simulink model "cddDcmApp"  (R2022b)
settings: Solver=FixedStepDiscrete  StartTime=0.0  StopTime=10.0  FixedStep=0.02  RelTol=1e-3
undefined symbols (not defined in the file or in its folder): CCPFaultType, DcChlType, HFAChlType, IcuChannelType, MtrRoutStaEnum, P_SignalPartType, P_Std_ReturnType, PowerChlType, SrsAdChlType, VGAChlType
(part 13 of 13)

== system cddDcmApp/DataServices_DID_D986_Vent_Grill_Actuator12_Second_Right_Y_Flap_DID_D986_2_No_Of_Movements/DataServices_DID_D986_Vent_Grill_Actuator12_Second_Right_Y_Flap_DID_D986_2_No_Of_Movements_WriteData_sys ==
  function : TriggerPort  TriggerType=function-call  IsSimulinkFunction=on  FunctionName=DataServices_DID_D986_Vent_Grill_Actuator12_Second_Right_Y_Flap_DID_D986_2_No_Of_Movements_WriteData  VariantControl=(inherit)
  Ground_1 : Ground
  Ground_2 : Ground
  Terminator_1 : Terminator
  Terminator_2 : Terminator
  Data : ArgIn  OutDataTypeStr=uint8  PortDimensions=4  ArgumentName=Data
  OpStatus : ArgIn  Port=2  OutDataTypeStr=Enum: Dcm_OpStatusType  ArgumentName=OpStatus
  ErrorCode : ArgOut  OutDataTypeStr=Enum: Dcm_NegativeResponseCodeType  ArgumentName=ErrorCode
  ERR : ArgOut  Port=2  OutDataTypeStr=Std_ReturnType  ArgumentName=ERR
  wires:
  Data/1 -> Terminator_1/1
  OpStatus/1 -> Terminator_2/1
  Ground_1/1 -> ErrorCode/1
  Ground_2/1 -> ERR/1

== system cddDcmApp/DataServices_DID_D987_Vent_Grill_Actuator12_Second_Right_Y_Flap_position_ComplexStructure_2/DataServices_DID_D987_Vent_Grill_Actuator12_Second_Right_Y_Flap_position_ComplexStructure_2_ConditionCheckRead_sys ==
  function : TriggerPort  TriggerType=function-call  IsSimulinkFunction=on  FunctionName=DataServices_DID_D987_Vent_Grill_Actuator12_Second_Right_Y_Flap_position_ComplexStructure_2_ConditionCheckRead  VariantControl=(inherit)
  Ground_1 : Ground
  Ground_2 : Ground
  ErrorCode : ArgOut  OutDataTypeStr=Enum: Dcm_NegativeResponseCodeType  ArgumentName=ErrorCode
  ERR : ArgOut  Port=2  OutDataTypeStr=Std_ReturnType  ArgumentName=ERR
  wires:
  Ground_1/1 -> ErrorCode/1
  Ground_2/1 -> ERR/1

== system cddDcmApp/DataServices_DID_D987_Vent_Grill_Actuator12_Second_Right_Y_Flap_position_ComplexStructure_2/DataServices_DID_D987_Vent_Grill_Actuator12_Second_Right_Y_Flap_position_ComplexStructure_2_ReadData_sys ==
  function : TriggerPort  TriggerType=function-call  IsSimulinkFunction=on  FunctionName=DataServices_DID_D987_Vent_Grill_Actuator12_Second_Right_Y_Flap_position_ComplexStructure_2_ReadData  VariantControl=(inherit)
  Ground_1 : Ground
  Ground_2 : Ground
  Data : ArgOut  OutDataTypeStr=uint8  ArgumentName=Data
  ERR : ArgOut  Port=2  OutDataTypeStr=Std_ReturnType  ArgumentName=ERR
  wires:
  Ground_1/1 -> Data/1
  Ground_2/1 -> ERR/1

== system cddDcmApp/DataServices_DID_D987_Vent_Grill_Actuator12_Second_Right_Y_Flap_position_DID_D987_1_Requested_Position/DataServices_DID_D987_Vent_Grill_Actuator12_Second_Right_Y_Flap_position_DID_D987_1_Requested_Position_ConditionCheckRead_sys ==
  function : TriggerPort  TriggerType=function-call  IsSimulinkFunction=on  FunctionName=<binary blob, 121 chars, not shown>  VariantControl=(inherit)
  Ground_1 : Ground
  Ground_2 : Ground
  ErrorCode : ArgOut  OutDataTypeStr=Enum: Dcm_NegativeResponseCodeType  ArgumentName=ErrorCode
  ERR : ArgOut  Port=2  OutDataTypeStr=Std_ReturnType  ArgumentName=ERR
  wires:
  Ground_1/1 -> ErrorCode/1
  Ground_2/1 -> ERR/1

== system cddDcmApp/DataServices_DID_D987_Vent_Grill_Actuator12_Second_Right_Y_Flap_position_DID_D987_1_Requested_Position/DataServices_DID_D987_Vent_Grill_Actuator12_Second_Right_Y_Flap_position_DID_D987_1_Requested_Position_ReadData_sys ==
  function : TriggerPort  TriggerType=function-call  IsSimulinkFunction=on  FunctionName=DataServices_DID_D987_Vent_Grill_Actuator12_Second_Right_Y_Flap_position_DID_D987_1_Requested_Position_ReadData  VariantControl=(inherit)
  Ground_1 : Ground
  Ground_2 : Ground
  Data : ArgOut  OutDataTypeStr=uint8  ArgumentName=Data
  ERR : ArgOut  Port=2  OutDataTypeStr=Std_ReturnType  ArgumentName=ERR
  wires:
  Ground_1/1 -> Data/1
  Ground_2/1 -> ERR/1

== system cddDcmApp/DataServices_DID_D987_Vent_Grill_Actuator12_Second_Right_Y_Flap_position_DID_D987_2_Actual_Position/DataServices_DID_D987_Vent_Grill_Actuator12_Second_Right_Y_Flap_position_DID_D987_2_Actual_Position_ConditionCheckRead_sys ==
  function : TriggerPort  TriggerType=function-call  IsSimulinkFunction=on  FunctionName=DataServices_DID_D987_Vent_Grill_Actuator12_Second_Right_Y_Flap_position_DID_D987_2_Actual_Position_ConditionCheckRead  VariantControl=(inherit)
  Ground_1 : Ground
  Ground_2 : Ground
  ErrorCode : ArgOut  OutDataTypeStr=Enum: Dcm_NegativeResponseCodeType  ArgumentName=ErrorCode
  ERR : ArgOut  Port=2  OutDataTypeStr=Std_ReturnType  ArgumentName=ERR
  wires:
  Ground_1/1 -> ErrorCode/1
  Ground_2/1 -> ERR/1

== system cddDcmApp/DataServices_DID_D987_Vent_Grill_Actuator12_Second_Right_Y_Flap_position_DID_D987_2_Actual_Position/DataServices_DID_D987_Vent_Grill_Actuator12_Second_Right_Y_Flap_position_DID_D987_2_Actual_Position_ReadData_sys ==
  function : TriggerPort  TriggerType=function-call  IsSimulinkFunction=on  FunctionName=DataServices_DID_D987_Vent_Grill_Actuator12_Second_Right_Y_Flap_position_DID_D987_2_Actual_Position_ReadData  VariantControl=(inherit)
  Ground_1 : Ground
  Ground_2 : Ground
  Data : ArgOut  OutDataTypeStr=uint8  ArgumentName=Data
  ERR : ArgOut  Port=2  OutDataTypeStr=Std_ReturnType  ArgumentName=ERR
  wires:
  Ground_1/1 -> Data/1
  Ground_2/1 -> ERR/1

== system cddDcmApp/DataServices_DID_D988_Vent_Grill_Actuator13_Second_Right_Y_Flap_DID_D988_1_step_Range/DataServices_DID_D988_Vent_Grill_Actuator13_Second_Right_Y_Flap_DID_D988_1_step_Range_ReadData_sys ==
  function : TriggerPort  TriggerType=function-call  IsSimulinkFunction=on  FunctionName=DataServices_DID_D988_Vent_Grill_Actuator13_Second_Right_Y_Flap_DID_D988_1_step_Range_ReadData  VariantControl=(inherit)
  Ground_1 : Ground
  Ground_2 : Ground
  Data : ArgOut  OutDataTypeStr=uint8  ArgumentName=Data
  ERR : ArgOut  Port=2  OutDataTypeStr=Std_ReturnType  ArgumentName=ERR
  wires:
  Ground_1/1 -> Data/1
  Ground_2/1 -> ERR/1

== system cddDcmApp/DataServices_DID_D988_Vent_Grill_Actuator13_Second_Right_Y_Flap_DID_D988_1_step_Range/DataServices_DID_D988_Vent_Grill_Actuator13_Second_Right_Y_Flap_DID_D988_1_step_Range_WriteData_sys ==
  function : TriggerPort  TriggerType=function-call  IsSimulinkFunction=on  FunctionName=DataServices_DID_D988_Vent_Grill_Actuator13_Second_Right_Y_Flap_DID_D988_1_step_Range_WriteData  VariantControl=(inherit)
  Ground_1 : Ground
  Ground_2 : Ground
  Terminator_1 : Terminator
  Data : ArgIn  OutDataTypeStr=uint8  PortDimensions=2  ArgumentName=Data
  ErrorCode : ArgOut  OutDataTypeStr=Enum: Dcm_NegativeResponseCodeType  ArgumentName=ErrorCode
  ERR : ArgOut  Port=2  OutDataTypeStr=Std_ReturnType  ArgumentName=ERR
  wires:
  Data/1 -> Terminator_1/1
  Ground_1/1 -> ErrorCode/1
  Ground_2/1 -> ERR/1

== system cddDcmApp/DataServices_DID_D988_Vent_Grill_Actuator13_Second_Right_Y_Flap_DID_D988_2_No_Of_Movements/DataServices_DID_D988_Vent_Grill_Actuator13_Second_Right_Y_Flap_DID_D988_2_No_Of_Movements_ReadData_sys ==
  function : TriggerPort  TriggerType=function-call  IsSimulinkFunction=on  FunctionName=DataServices_DID_D988_Vent_Grill_Actuator13_Second_Right_Y_Flap_DID_D988_2_No_Of_Movements_ReadData  VariantControl=(inherit)
  Ground_1 : Ground
  Ground_2 : Ground
  Data : ArgOut  OutDataTypeStr=uint8  ArgumentName=Data
  ERR : ArgOut  Port=2  OutDataTypeStr=Std_ReturnType  ArgumentName=ERR
  wires:
  Ground_1/1 -> Data/1
  Ground_2/1 -> ERR/1

== system cddDcmApp/DataServices_DID_D988_Vent_Grill_Actuator13_Second_Right_Y_Flap_DID_D988_2_No_Of_Movements/DataServices_DID_D988_Vent_Grill_Actuator13_Second_Right_Y_Flap_DID_D988_2_No_Of_Movements_WriteData_sys ==
  function : TriggerPort  TriggerType=function-call  IsSimulinkFunction=on  FunctionName=DataServices_DID_D988_Vent_Grill_Actuator13_Second_Right_Y_Flap_DID_D988_2_No_Of_Movements_WriteData  VariantControl=(inherit)
  Ground_1 : Ground
  Ground_2 : Ground
  Terminator_1 : Terminator
  Data : ArgIn  OutDataTypeStr=uint8  PortDimensions=4  ArgumentName=Data
  ErrorCode : ArgOut  OutDataTypeStr=Enum: Dcm_NegativeResponseCodeType  ArgumentName=ErrorCode
  ERR : ArgOut  Port=2  OutDataTypeStr=Std_ReturnType  ArgumentName=ERR
  wires:
  Data/1 -> Terminator_1/1
  Ground_1/1 -> ErrorCode/1
  Ground_2/1 -> ERR/1

== system cddDcmApp/DataServices_DID_D989_Vent_Grill_Actuator13_Second_Right_Y_Flap_position_DID_D989_1_Requested_Position/DataServices_DID_D989_Vent_Grill_Actuator13_Second_Right_Y_Flap_position_DID_D989_1_Requested_Position_ReadData_sys ==
  function : TriggerPort  TriggerType=function-call  IsSimulinkFunction=on  FunctionName=DataServices_DID_D989_Vent_Grill_Actuator13_Second_Right_Y_Flap_position_DID_D989_1_Requested_Position_ReadData  VariantControl=(inherit)
  Ground_1 : Ground
  Ground_2 : Ground
  Data : ArgOut  OutDataTypeStr=uint8  ArgumentName=Data
  ERR : ArgOut  Port=2  OutDataTypeStr=Std_ReturnType  ArgumentName=ERR
  wires:
  Ground_1/1 -> Data/1
  Ground_2/1 -> ERR/1

== system cddDcmApp/DataServices_DID_D989_Vent_Grill_Actuator13_Second_Right_Y_Flap_position_DID_D989_2_Actual_Position/DataServices_DID_D989_Vent_Grill_Actuator13_Second_Right_Y_Flap_position_DID_D989_2_Actual_Position_ReadData_sys ==
  function : TriggerPort  TriggerType=function-call  IsSimulinkFunction=on  FunctionName=DataServices_DID_D989_Vent_Grill_Actuator13_Second_Right_Y_Flap_position_DID_D989_2_Actual_Position_ReadData  VariantControl=(inherit)
  Ground_1 : Ground
  Ground_2 : Ground
  Data : ArgOut  OutDataTypeStr=uint8  ArgumentName=Data
  ERR : ArgOut  Port=2  OutDataTypeStr=Std_ReturnType  ArgumentName=ERR
  wires:
  Ground_1/1 -> Data/1
  Ground_2/1 -> ERR/1

== system cddDcmApp/DataServices_DID_D989_Vent_Grill_Actuator13_Second_Right_Y_Flap_position_DID_D989_3_Calibration_status/DataServices_DID_D989_Vent_Grill_Actuator13_Second_Right_Y_Flap_position_DID_D989_3_Calibration_status_ReadData_sys ==
  function : TriggerPort  TriggerType=function-call  IsSimulinkFunction=on  FunctionName=DataServices_DID_D989_Vent_Grill_Actuator13_Second_Right_Y_Flap_position_DID_D989_3_Calibration_status_ReadData  VariantControl=(inherit)
  Ground_1 : Ground
  Ground_2 : Ground
  Data : ArgOut  OutDataTypeStr=uint8  ArgumentName=Data
  ERR : ArgOut  Port=2  OutDataTypeStr=Std_ReturnType  ArgumentName=ERR
  wires:
  Ground_1/1 -> Data/1
  Ground_2/1 -> ERR/1

== system cddDcmApp/DataServices_DID_D989_Vent_Grill_Actuator13_Second_Right_Y_Flap_position_DID_D989_4_Working_Status/DataServices_DID_D989_Vent_Grill_Actuator13_Second_Right_Y_Flap_position_DID_D989_4_Working_Status_ReadData_sys ==
  function : TriggerPort  TriggerType=function-call  IsSimulinkFunction=on  FunctionName=DataServices_DID_D989_Vent_Grill_Actuator13_Second_Right_Y_Flap_position_DID_D989_4_Working_Status_ReadData  VariantControl=(inherit)
  Ground_1 : Ground
  Ground_2 : Ground
  Data : ArgOut  OutDataTypeStr=uint8  ArgumentName=Data
  ERR : ArgOut  Port=2  OutDataTypeStr=Std_ReturnType  ArgumentName=ERR
  wires:
  Ground_1/1 -> Data/1
  Ground_2/1 -> ERR/1

== system cddDcmApp/DataServices_DID_D98A_Vent_Grill_Actuator14_Second_Right_Y_Flap_DID_D98A_1_step_Range/DataServices_DID_D98A_Vent_Grill_Actuator14_Second_Right_Y_Flap_DID_D98A_1_step_Range_ReadData_sys ==
  function : TriggerPort  TriggerType=function-call  IsSimulinkFunction=on  FunctionName=DataServices_DID_D98A_Vent_Grill_Actuator14_Second_Right_Y_Flap_DID_D98A_1_step_Range_ReadData  VariantControl=(inherit)
  Ground_1 : Ground
  Ground_2 : Ground
  Data : ArgOut  OutDataTypeStr=uint8  ArgumentName=Data
  ERR : ArgOut  Port=2  OutDataTypeStr=Std_ReturnType  ArgumentName=ERR
  wires:
  Ground_1/1 -> Data/1
  Ground_2/1 -> ERR/1

== system cddDcmApp/DataServices_DID_D98A_Vent_Grill_Actuator14_Second_Right_Y_Flap_DID_D98A_1_step_Range/DataServices_DID_D98A_Vent_Grill_Actuator14_Second_Right_Y_Flap_DID_D98A_1_step_Range_WriteData_sys ==
  function : TriggerPort  TriggerType=function-call  IsSimulinkFunction=on  FunctionName=DataServices_DID_D98A_Vent_Grill_Actuator14_Second_Right_Y_Flap_DID_D98A_1_step_Range_WriteData  VariantControl=(inherit)
  Ground_1 : Ground
  Ground_2 : Ground
  Terminator_1 : Terminator
  Data : ArgIn  OutDataTypeStr=uint8  PortDimensions=2  ArgumentName=Data
  ErrorCode : ArgOut  OutDataTypeStr=Enum: Dcm_NegativeResponseCodeType  ArgumentName=ErrorCode
  ERR : ArgOut  Port=2  OutDataTypeStr=Std_ReturnType  ArgumentName=ERR
  wires:
  Data/1 -> Terminator_1/1
  Ground_1/1 -> ErrorCode/1
  Ground_2/1 -> ERR/1

== system cddDcmApp/DataServices_DID_D98A_Vent_Grill_Actuator14_Second_Right_Y_Flap_DID_D98A_2_No_Of_Movements/DataServices_DID_D98A_Vent_Grill_Actuator14_Second_Right_Y_Flap_DID_D98A_2_No_Of_Movements_ReadData_sys ==
  function : TriggerPort  TriggerType=function-call  IsSimulinkFunction=on  FunctionName=DataServices_DID_D98A_Vent_Grill_Actuator14_Second_Right_Y_Flap_DID_D98A_2_No_Of_Movements_ReadData  VariantControl=(inherit)
  Ground_1 : Ground
  Ground_2 : Ground
  Data : ArgOut  OutDataTypeStr=uint8  ArgumentName=Data
  ERR : ArgOut  Port=2  OutDataTypeStr=Std_ReturnType  ArgumentName=ERR
  wires:
  Ground_1/1 -> Data/1
  Ground_2/1 -> ERR/1

== system cddDcmApp/DataServices_DID_D98A_Vent_Grill_Actuator14_Second_Right_Y_Flap_DID_D98A_2_No_Of_Movements/DataServices_DID_D98A_Vent_Grill_Actuator14_Second_Right_Y_Flap_DID_D98A_2_No_Of_Movements_WriteData_sys ==
  function : TriggerPort  TriggerType=function-call  IsSimulinkFunction=on  FunctionName=DataServices_DID_D98A_Vent_Grill_Actuator14_Second_Right_Y_Flap_DID_D98A_2_No_Of_Movements_WriteData  VariantControl=(inherit)
  Ground_1 : Ground
  Ground_2 : Ground
  Terminator_1 : Terminator
  Data : ArgIn  OutDataTypeStr=uint8  PortDimensions=4  ArgumentName=Data
  ErrorCode : ArgOut  OutDataTypeStr=Enum: Dcm_NegativeResponseCodeType  ArgumentName=ErrorCode
  ERR : ArgOut  Port=2  OutDataTypeStr=Std_ReturnType  ArgumentName=ERR
  wires:
  Data/1 -> Terminator_1/1
  Ground_1/1 -> ErrorCode/1
  Ground_2/1 -> ERR/1

== system cddDcmApp/DataServices_DID_D98B_Vent_Grill_Actuator14_Second_Right_Y_Flap_position_DID_D98B_1_Requested_Position/DataServices_DID_D98B_Vent_Grill_Actuator14_Second_Right_Y_Flap_position_DID_D98B_1_Requested_Position_ReadData_sys ==
  function : TriggerPort  TriggerType=function-call  IsSimulinkFunction=on  FunctionName=DataServices_DID_D98B_Vent_Grill_Actuator14_Second_Right_Y_Flap_position_DID_D98B_1_Requested_Position_ReadData  VariantControl=(inherit)
  Ground_1 : Ground
  Ground_2 : Ground
  Data : ArgOut  OutDataTypeStr=uint8  ArgumentName=Data
  ERR : ArgOut  Port=2  OutDataTypeStr=Std_ReturnType  ArgumentName=ERR
  wires:
  Ground_1/1 -> Data/1
  Ground_2/1 -> ERR/1

== system cddDcmApp/DataServices_DID_D98B_Vent_Grill_Actuator14_Second_Right_Y_Flap_position_DID_D98B_2_Actual_Position/DataServices_DID_D98B_Vent_Grill_Actuator14_Second_Right_Y_Flap_position_DID_D98B_2_Actual_Position_ReadData_sys ==
  function : TriggerPort  TriggerType=function-call  IsSimulinkFunction=on  FunctionName=DataServices_DID_D98B_Vent_Grill_Actuator14_Second_Right_Y_Flap_position_DID_D98B_2_Actual_Position_ReadData  VariantControl=(inherit)
  Ground_1 : Ground
  Ground_2 : Ground
  Data : ArgOut  OutDataTypeStr=uint8  ArgumentName=Data
  ERR : ArgOut  Port=2  OutDataTypeStr=Std_ReturnType  ArgumentName=ERR
  wires:
  Ground_1/1 -> Data/1
  Ground_2/1 -> ERR/1

== system cddDcmApp/DataServices_DID_D98B_Vent_Grill_Actuator14_Second_Right_Y_Flap_position_DID_D98B_3_Calibration_Status/DataServices_DID_D98B_Vent_Grill_Actuator14_Second_Right_Y_Flap_position_DID_D98B_3_Calibration_Status_ReadData_sys ==
  function : TriggerPort  TriggerType=function-call  IsSimulinkFunction=on  FunctionName=DataServices_DID_D98B_Vent_Grill_Actuator14_Second_Right_Y_Flap_position_DID_D98B_3_Calibration_Status_ReadData  VariantControl=(inherit)
  Ground_1 : Ground
  Ground_2 : Ground
  Data : ArgOut  OutDataTypeStr=uint8  ArgumentName=Data
  ERR : ArgOut  Port=2  OutDataTypeStr=Std_ReturnType  ArgumentName=ERR
  wires:
  Ground_1/1 -> Data/1
  Ground_2/1 -> ERR/1

== system cddDcmApp/DataServices_DID_D98B_Vent_Grill_Actuator14_Second_Right_Y_Flap_position_DID_D98B_4_Working_Status/DataServices_DID_D98B_Vent_Grill_Actuator14_Second_Right_Y_Flap_position_DID_D98B_4_Working_Status_ReadData_sys ==
  function : TriggerPort  TriggerType=function-call  IsSimulinkFunction=on  FunctionName=DataServices_DID_D98B_Vent_Grill_Actuator14_Second_Right_Y_Flap_position_DID_D98B_4_Working_Status_ReadData  VariantControl=(inherit)
  Ground_1 : Ground
  Ground_2 : Ground
  Data : ArgOut  OutDataTypeStr=uint8  ArgumentName=Data
  ERR : ArgOut  Port=2  OutDataTypeStr=Std_ReturnType  ArgumentName=ERR
  wires:
  Ground_1/1 -> Data/1
  Ground_2/1 -> ERR/1

== system cddDcmApp/DataServices_DID_D98C_Vent_Grill_Actuator15_Second_Right_Y_Flap_DID_D98C_1_step_Range/DataServices_DID_D98C_Vent_Grill_Actuator15_Second_Right_Y_Flap_DID_D98C_1_step_Range_ReadData_sys ==
  function : TriggerPort  TriggerType=function-call  IsSimulinkFunction=on  FunctionName=DataServices_DID_D98C_Vent_Grill_Actuator15_Second_Right_Y_Flap_DID_D98C_1_step_Range_ReadData  VariantControl=(inherit)
  Ground_1 : Ground
  Ground_2 : Ground
  Data : ArgOut  OutDataTypeStr=uint8  ArgumentName=Data
  ERR : ArgOut  Port=2  OutDataTypeStr=Std_ReturnType  ArgumentName=ERR
  wires:
  Ground_1/1 -> Data/1
  Ground_2/1 -> ERR/1

== system cddDcmApp/DataServices_DID_D98C_Vent_Grill_Actuator15_Second_Right_Y_Flap_DID_D98C_1_step_Range/DataServices_DID_D98C_Vent_Grill_Actuator15_Second_Right_Y_Flap_DID_D98C_1_step_Range_WriteData_sys ==
  function : TriggerPort  TriggerType=function-call  IsSimulinkFunction=on  FunctionName=DataServices_DID_D98C_Vent_Grill_Actuator15_Second_Right_Y_Flap_DID_D98C_1_step_Range_WriteData  VariantControl=(inherit)
  Ground_1 : Ground
  Ground_2 : Ground
  Terminator_1 : Terminator
  Data : ArgIn  OutDataTypeStr=uint8  PortDimensions=2  ArgumentName=Data
  ErrorCode : ArgOut  OutDataTypeStr=Enum: Dcm_NegativeResponseCodeType  ArgumentName=ErrorCode
  ERR : ArgOut  Port=2  OutDataTypeStr=Std_ReturnType  ArgumentName=ERR
  wires:
  Data/1 -> Terminator_1/1
  Ground_1/1 -> ErrorCode/1
  Ground_2/1 -> ERR/1

== system cddDcmApp/DataServices_DID_D98C_Vent_Grill_Actuator15_Second_Right_Y_Flap_DID_D98C_2_No_Of_Movements/DataServices_DID_D98C_Vent_Grill_Actuator15_Second_Right_Y_Flap_DID_D98C_2_No_Of_Movements_ReadData_sys ==
  function : TriggerPort  TriggerType=function-call  IsSimulinkFunction=on  FunctionName=DataServices_DID_D98C_Vent_Grill_Actuator15_Second_Right_Y_Flap_DID_D98C_2_No_Of_Movements_ReadData  VariantControl=(inherit)
  Ground_1 : Ground
  Ground_2 : Ground
  Data : ArgOut  OutDataTypeStr=uint8  ArgumentName=Data
  ERR : ArgOut  Port=2  OutDataTypeStr=Std_ReturnType  ArgumentName=ERR
  wires:
  Ground_1/1 -> Data/1
  Ground_2/1 -> ERR/1

== system cddDcmApp/DataServices_DID_D98C_Vent_Grill_Actuator15_Second_Right_Y_Flap_DID_D98C_2_No_Of_Movements/DataServices_DID_D98C_Vent_Grill_Actuator15_Second_Right_Y_Flap_DID_D98C_2_No_Of_Movements_WriteData_sys ==
  function : TriggerPort  TriggerType=function-call  IsSimulinkFunction=on  FunctionName=DataServices_DID_D98C_Vent_Grill_Actuator15_Second_Right_Y_Flap_DID_D98C_2_No_Of_Movements_WriteData  VariantControl=(inherit)
  Ground_1 : Ground
  Ground_2 : Ground
  Terminator_1 : Terminator
  Data : ArgIn  OutDataTypeStr=uint8  PortDimensions=4  ArgumentName=Data
  ErrorCode : ArgOut  OutDataTypeStr=Enum: Dcm_NegativeResponseCodeType  ArgumentName=ErrorCode
  ERR : ArgOut  Port=2  OutDataTypeStr=Std_ReturnType  ArgumentName=ERR
  wires:
  Data/1 -> Terminator_1/1
  Ground_1/1 -> ErrorCode/1
  Ground_2/1 -> ERR/1

== system cddDcmApp/DataServices_DID_D98D_Vent_Grill_Actuator15_Second_Right_Y_Flap_position_ComplexStructure_2/DataServices_DID_D98D_Vent_Grill_Actuator15_Second_Right_Y_Flap_position_ComplexStructure_2_ReadData_sys ==
  function : TriggerPort  TriggerType=function-call  IsSimulinkFunction=on  FunctionName=DataServices_DID_D98D_Vent_Grill_Actuator15_Second_Right_Y_Flap_position_ComplexStructure_2_ReadData  VariantControl=(inherit)
  Ground_1 : Ground
  Ground_2 : Ground
  Data : ArgOut  OutDataTypeStr=uint8  ArgumentName=Data
  ERR : ArgOut  Port=2  OutDataTypeStr=Std_ReturnType  ArgumentName=ERR
  wires:
  Ground_1/1 -> Data/1
  Ground_2/1 -> ERR/1

== system cddDcmApp/DataServices_DID_D98D_Vent_Grill_Actuator15_Second_Right_Y_Flap_position_DID_D98D_1_Requested_Position/DataServices_DID_D98D_Vent_Grill_Actuator15_Second_Right_Y_Flap_position_DID_D98D_1_Requested_Position_ReadData_sys ==
  function : TriggerPort  TriggerType=function-call  IsSimulinkFunction=on  FunctionName=DataServices_DID_D98D_Vent_Grill_Actuator15_Second_Right_Y_Flap_position_DID_D98D_1_Requested_Position_ReadData  VariantControl=(inherit)
  Ground_1 : Ground
  Ground_2 : Ground
  Data : ArgOut  OutDataTypeStr=uint8  ArgumentName=Data
  ERR : ArgOut  Port=2  OutDataTypeStr=Std_ReturnType  ArgumentName=ERR
  wires:
  Ground_1/1 -> Data/1
  Ground_2/1 -> ERR/1

== system cddDcmApp/DataServices_DID_D98D_Vent_Grill_Actuator15_Second_Right_Y_Flap_position_DID_D98D_2_Actual_Position/DataServices_DID_D98D_Vent_Grill_Actuator15_Second_Right_Y_Flap_position_DID_D98D_2_Actual_Position_ReadData_sys ==
  function : TriggerPort  TriggerType=function-call  IsSimulinkFunction=on  FunctionName=DataServices_DID_D98D_Vent_Grill_Actuator15_Second_Right_Y_Flap_position_DID_D98D_2_Actual_Position_ReadData  VariantControl=(inherit)
  Ground_1 : Ground
  Ground_2 : Ground
  Data : ArgOut  OutDataTypeStr=uint8  ArgumentName=Data
  ERR : ArgOut  Port=2  OutDataTypeStr=Std_ReturnType  ArgumentName=ERR
  wires:
  Ground_1/1 -> Data/1
  Ground_2/1 -> ERR/1

== system cddDcmApp/DataServices_DID_D98E_Vent_Grill_Actuator16_Second_Right_Y_Flap_DID_D98E_1_step_Range/DataServices_DID_D98E_Vent_Grill_Actuator16_Second_Right_Y_Flap_DID_D98E_1_step_Range_ReadData_sys ==
  function : TriggerPort  TriggerType=function-call  IsSimulinkFunction=on  FunctionName=DataServices_DID_D98E_Vent_Grill_Actuator16_Second_Right_Y_Flap_DID_D98E_1_step_Range_ReadData  VariantControl=(inherit)
  Ground_1 : Ground
  Ground_2 : Ground
  Data : ArgOut  OutDataTypeStr=uint8  ArgumentName=Data
  ERR : ArgOut  Port=2  OutDataTypeStr=Std_ReturnType  ArgumentName=ERR
  wires:
  Ground_1/1 -> Data/1
  Ground_2/1 -> ERR/1

== system cddDcmApp/DataServices_DID_D98E_Vent_Grill_Actuator16_Second_Right_Y_Flap_DID_D98E_1_step_Range/DataServices_DID_D98E_Vent_Grill_Actuator16_Second_Right_Y_Flap_DID_D98E_1_step_Range_WriteData_sys ==
  function : TriggerPort  TriggerType=function-call  IsSimulinkFunction=on  FunctionName=DataServices_DID_D98E_Vent_Grill_Actuator16_Second_Right_Y_Flap_DID_D98E_1_step_Range_WriteData  VariantControl=(inherit)
  Ground_1 : Ground
  Ground_2 : Ground
  Terminator_1 : Terminator
  Data : ArgIn  OutDataTypeStr=uint8  PortDimensions=2  ArgumentName=Data
  ErrorCode : ArgOut  OutDataTypeStr=Enum: Dcm_NegativeResponseCodeType  ArgumentName=ErrorCode
  ERR : ArgOut  Port=2  OutDataTypeStr=Std_ReturnType  ArgumentName=ERR
  wires:
  Data/1 -> Terminator_1/1
  Ground_1/1 -> ErrorCode/1
  Ground_2/1 -> ERR/1

== system cddDcmApp/DataServices_DID_D98E_Vent_Grill_Actuator16_Second_Right_Y_Flap_DID_D98E_2_No_Of_Movements/DataServices_DID_D98E_Vent_Grill_Actuator16_Second_Right_Y_Flap_DID_D98E_2_No_Of_Movements_ReadData_sys ==
  function : TriggerPort  TriggerType=function-call  IsSimulinkFunction=on  FunctionName=DataServices_DID_D98E_Vent_Grill_Actuator16_Second_Right_Y_Flap_DID_D98E_2_No_Of_Movements_ReadData  VariantControl=(inherit)
  Ground_1 : Ground
  Ground_2 : Ground
  Data : ArgOut  OutDataTypeStr=uint8  ArgumentName=Data
  ERR : ArgOut  Port=2  OutDataTypeStr=Std_ReturnType  ArgumentName=ERR
  wires:
  Ground_1/1 -> Data/1
  Ground_2/1 -> ERR/1

== system cddDcmApp/DataServices_DID_D98E_Vent_Grill_Actuator16_Second_Right_Y_Flap_DID_D98E_2_No_Of_Movements/DataServices_DID_D98E_Vent_Grill_Actuator16_Second_Right_Y_Flap_DID_D98E_2_No_Of_Movements_WriteData_sys ==
  function : TriggerPort  TriggerType=function-call  IsSimulinkFunction=on  FunctionName=DataServices_DID_D98E_Vent_Grill_Actuator16_Second_Right_Y_Flap_DID_D98E_2_No_Of_Movements_WriteData  VariantControl=(inherit)
  Ground_1 : Ground
  Ground_2 : Ground
  Terminator_1 : Terminator
  Data : ArgIn  OutDataTypeStr=uint8  PortDimensions=4  ArgumentName=Data
  ErrorCode : ArgOut  OutDataTypeStr=Enum: Dcm_NegativeResponseCodeType  ArgumentName=ErrorCode
  ERR : ArgOut  Port=2  OutDataTypeStr=Std_ReturnType  ArgumentName=ERR
  wires:
  Data/1 -> Terminator_1/1
  Ground_1/1 -> ErrorCode/1
  Ground_2/1 -> ERR/1

== system cddDcmApp/DataServices_DID_D98F_Vent_Grill_Actuator16_Second_Right_Y_Flap_position_ComplexStructure_2/DataServices_DID_D98F_Vent_Grill_Actuator16_Second_Right_Y_Flap_position_ComplexStructure_2_ReadData_sys ==
  function : TriggerPort  TriggerType=function-call  IsSimulinkFunction=on  FunctionName=DataServices_DID_D98F_Vent_Grill_Actuator16_Second_Right_Y_Flap_position_ComplexStructure_2_ReadData  VariantControl=(inherit)
  Ground_1 : Ground
  Ground_2 : Ground
  Data : ArgOut  OutDataTypeStr=uint8  ArgumentName=Data
  ERR : ArgOut  Port=2  OutDataTypeStr=Std_ReturnType  ArgumentName=ERR
  wires:
  Ground_1/1 -> Data/1
  Ground_2/1 -> ERR/1

== system cddDcmApp/DataServices_DID_D98F_Vent_Grill_Actuator16_Second_Right_Y_Flap_position_DID_D98F_1_Requested_Position/DataServices_DID_D98F_Vent_Grill_Actuator16_Second_Right_Y_Flap_position_DID_D98F_1_Requested_Position_ReadData_sys ==
  function : TriggerPort  TriggerType=function-call  IsSimulinkFunction=on  FunctionName=DataServices_DID_D98F_Vent_Grill_Actuator16_Second_Right_Y_Flap_position_DID_D98F_1_Requested_Position_ReadData  VariantControl=(inherit)
  Ground_1 : Ground
  Ground_2 : Ground
  Data : ArgOut  OutDataTypeStr=uint8  ArgumentName=Data
  ERR : ArgOut  Port=2  OutDataTypeStr=Std_ReturnType  ArgumentName=ERR
  wires:
  Ground_1/1 -> Data/1
  Ground_2/1 -> ERR/1

== system cddDcmApp/DataServices_DID_D98F_Vent_Grill_Actuator16_Second_Right_Y_Flap_position_DID_D98F_2_Actual_Position/DataServices_DID_D98F_Vent_Grill_Actuator16_Second_Right_Y_Flap_position_DID_D98F_2_Actual_Position_ReadData_sys ==
  function : TriggerPort  TriggerType=function-call  IsSimulinkFunction=on  FunctionName=DataServices_DID_D98F_Vent_Grill_Actuator16_Second_Right_Y_Flap_position_DID_D98F_2_Actual_Position_ReadData  VariantControl=(inherit)
  Ground_1 : Ground
  Ground_2 : Ground
  Data : ArgOut  OutDataTypeStr=uint8  ArgumentName=Data
  ERR : ArgOut  Port=2  OutDataTypeStr=Std_ReturnType  ArgumentName=ERR
  wires:
  Ground_1/1 -> Data/1
  Ground_2/1 -> ERR/1

== system cddDcmApp/DataServices_DID_DA00_DID_DA00_Read_CCM_Internal_Data_For/DataServices_DID_DA00_DID_DA00_Read_CCM_Internal_Data_For_Analysis_sys ==
  function : TriggerPort  TriggerType=function-call  IsSimulinkFunction=on  FunctionName=DataServices_DID_DA00_DID_DA00_Read_CCM_Internal_Data_For_Analysis  VariantControl=(inherit)
  Ground_1 : Ground
  Ground_2 : Ground
  Terminator_1 : Terminator
  OpStatus : ArgIn  OutDataTypeStr=Enum: Dcm_OpStatusType  ArgumentName=OpStatus
  Data : ArgOut  OutDataTypeStr=uint8  ArgumentName=Data
  ERR : ArgOut  Port=2  OutDataTypeStr=Std_ReturnType  ArgumentName=ERR
  wires:
  OpStatus/1 -> Terminator_1/1
  Ground_1/1 -> Data/1
  Ground_2/1 -> ERR/1

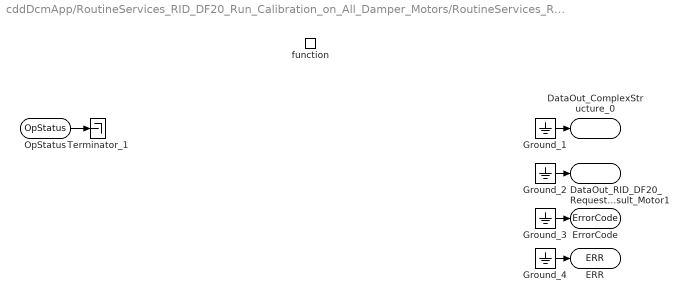
== system cddDcmApp/RoutineServices_RID_DF20_Run_Calibration_on_All_Damper_Motors/RoutineServices_RID_DF20_Run_Calibration_on_All_Damper_Motors_RequestResults_sys ==
  function : TriggerPort  TriggerType=function-call  IsSimulinkFunction=on  FunctionName=RoutineServices_RID_DF20_Run_Calibration_on_All_Damper_Motors_RequestResults  VariantControl=(inherit)
  Ground_{1..4} (4 alike) : Ground
  Terminator_1 : Terminator
  OpStatus : ArgIn  OutDataTypeStr=Enum: Dcm_OpStatusType  ArgumentName=OpStatus
  DataOut_ComplexStructure_0 : ArgOut  OutDataTypeStr=uint8  ArgumentName=DataOut_ComplexStructure_0
  DataOut_RID_DF20_RequestResult_Pr_3_Routinue_Result_Motor1 : ArgOut  Port=2  OutDataTypeStr=uint8  ArgumentName=DataOut_RID_DF20_RequestResult_Pr_3_Routinue_Result_Motor1
  ErrorCode : ArgOut  Port=3  OutDataTypeStr=Enum: Dcm_NegativeResponseCodeType  ArgumentName=ErrorCode
  ERR : ArgOut  Port=4  OutDataTypeStr=Std_ReturnType  ArgumentName=ERR
  wires:
  OpStatus/1 -> Terminator_1/1
  Ground_1/1 -> DataOut_ComplexStructure_0/1
  Ground_2/1 -> DataOut_RID_DF20_RequestResult_Pr_3_Routinue_Result_Motor1/1
  Ground_3/1 -> ErrorCode/1
  Ground_4/1 -> ERR/1

== system cddDcmApp/RoutineServices_RID_DF20_Run_Calibration_on_All_Damper_Motors/RoutineServices_RID_DF20_Run_Calibration_on_All_Damper_Motors_Start_sys ==
  function : TriggerPort  TriggerType=function-call  IsSimulinkFunction=on  FunctionName=RoutineServices_RID_DF20_Run_Calibration_on_All_Damper_Motors_Start  VariantControl=(inherit)
  Ground_1 : Ground
  Ground_2 : Ground
  Ground_3 : Ground
  Terminator_1 : Terminator
  OpStatus : ArgIn  OutDataTypeStr=Enum: Dcm_OpStatusType  ArgumentName=OpStatus
  DataOut_Routine_Data_Record : ArgOut  OutDataTypeStr=uint8  ArgumentName=DataOut_Routine_Data_Record
  ErrorCode : ArgOut  Port=2  OutDataTypeStr=Enum: Dcm_NegativeResponseCodeType  ArgumentName=ErrorCode
  ERR : ArgOut  Port=3  OutDataTypeStr=Std_ReturnType  ArgumentName=ERR
  wires:
  OpStatus/1 -> Terminator_1/1
  Ground_1/1 -> DataOut_Routine_Data_Record/1
  Ground_2/1 -> ErrorCode/1
  Ground_3/1 -> ERR/1

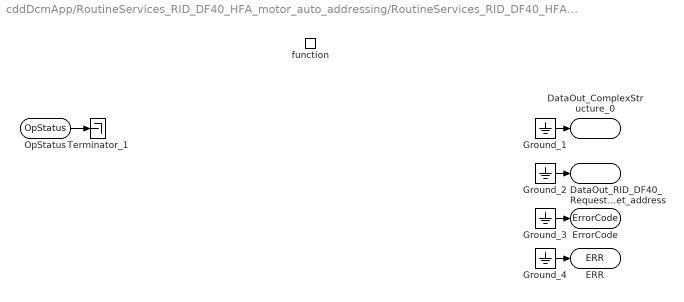
== system cddDcmApp/RoutineServices_RID_DF40_HFA_motor_auto_addressing/RoutineServices_RID_DF40_HFA_motor_auto_addressing_RequestResults_sys ==
  function : TriggerPort  TriggerType=function-call  IsSimulinkFunction=on  FunctionName=RoutineServices_RID_DF40_HFA_motor_auto_addressing_RequestResults  VariantControl=(inherit)
  Ground_{1..4} (4 alike) : Ground
  Terminator_1 : Terminator
  OpStatus : ArgIn  OutDataTypeStr=Enum: Dcm_OpStatusType  ArgumentName=OpStatus
  DataOut_ComplexStructure_0 : ArgOut  OutDataTypeStr=uint8  ArgumentName=DataOut_ComplexStructure_0
  DataOut_RID_DF40_RequestResult_Pr_3_Routinue_Result_set_address : ArgOut  Port=2  OutDataTypeStr=uint8  ArgumentName=DataOut_RID_DF40_RequestResult_Pr_3_Routinue_Result_set_address
  ErrorCode : ArgOut  Port=3  OutDataTypeStr=Enum: Dcm_NegativeResponseCodeType  ArgumentName=ErrorCode
  ERR : ArgOut  Port=4  OutDataTypeStr=Std_ReturnType  ArgumentName=ERR
  wires:
  OpStatus/1 -> Terminator_1/1
  Ground_1/1 -> DataOut_ComplexStructure_0/1
  Ground_2/1 -> DataOut_RID_DF40_RequestResult_Pr_3_Routinue_Result_set_address/1
  Ground_3/1 -> ErrorCode/1
  Ground_4/1 -> ERR/1

== system cddDcmApp/RoutineServices_RID_DF40_HFA_motor_auto_addressing/RoutineServices_RID_DF40_HFA_motor_auto_addressing_Start_sys ==
  function : TriggerPort  TriggerType=function-call  IsSimulinkFunction=on  FunctionName=RoutineServices_RID_DF40_HFA_motor_auto_addressing_Start  VariantControl=(inherit)
  Ground_1 : Ground
  Ground_2 : Ground
  Ground_3 : Ground
  Terminator_1 : Terminator
  OpStatus : ArgIn  OutDataTypeStr=Enum: Dcm_OpStatusType  ArgumentName=OpStatus
  DataOut_Routine_Data_Record : ArgOut  OutDataTypeStr=uint8  ArgumentName=DataOut_Routine_Data_Record
  ErrorCode : ArgOut  Port=2  OutDataTypeStr=Enum: Dcm_NegativeResponseCodeType  ArgumentName=ErrorCode
  ERR : ArgOut  Port=3  OutDataTypeStr=Std_ReturnType  ArgumentName=ERR
  wires:
  OpStatus/1 -> Terminator_1/1
  Ground_1/1 -> DataOut_Routine_Data_Record/1
  Ground_2/1 -> ErrorCode/1
  Ground_3/1 -> ERR/1
